simulink model "test03"  (R2022a)
settings: Solver=FixedStepAuto  StartTime=0.0  StopTime=0.05  FixedStep=1e-4  RelTol=1e-3
values from neighbouring files (symbols this model uses):
  A = 0.01    [parameter_load3.m]
  d = 2.6    [parameter_load3.m]
  I = 3055e-8    (= 3.055e-05)    [parameter_load3.m]
  jv = 1064400    [parameter_load3.m]
  kf = 6.67e7    (= 66700000)    [parameter_load3.m]
  kv = 400000    [parameter_load3.m]
  L = 210    [parameter_load3.m]
  m = 7800*(7684e-6)    (= 59.9352)    [parameter_load3.m]
  mv = 42400    [parameter_load3.m]
  position = 0.5*L    (= 105)    [parameter_load3.m]
  r = 840/1000/2    (= 0.42)    [parameter_load3.m]
  v = 100/3.6    (= 27.7777777778)    [parameter_load3.m]
(part 1 of 73)
settings Model: Creator=Spicydel  LastModifiedBy=Spicydel

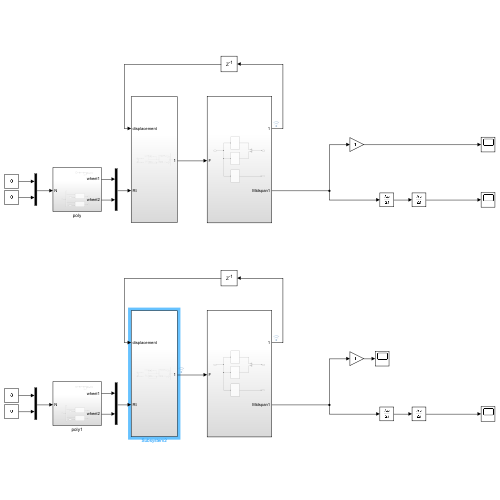
[thumbnail: Simulink's stored preview of the top-level diagram]
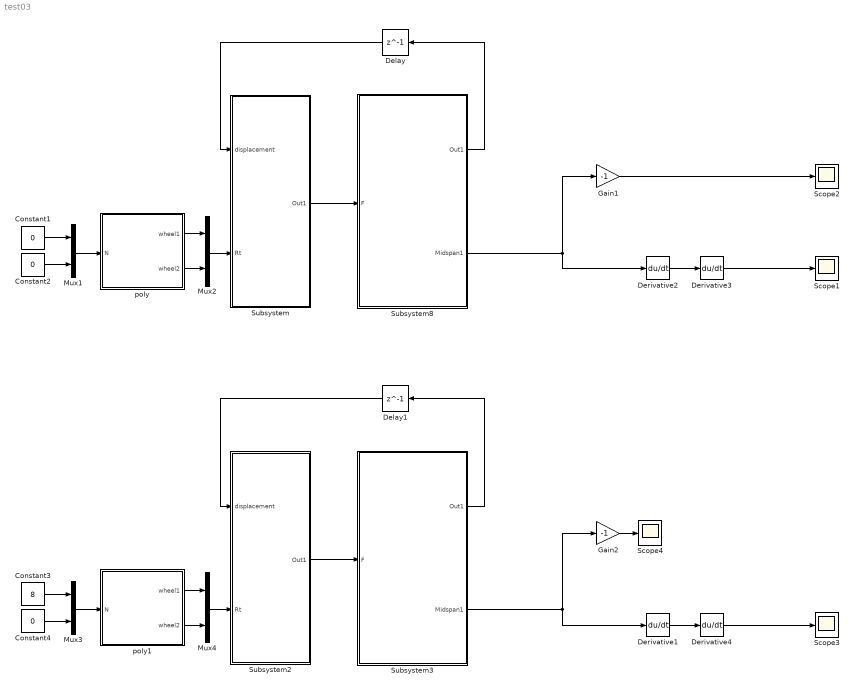
== system test03 ==
  Constant1 : Constant  Value=0
  Constant2 : Constant  Value=0
  Constant3 : Constant  Value=8
  Constant4 : Constant  Value=0
  Delay : Delay  DelayLength=1
  Delay1 : Delay  DelayLength=1
  Derivative{1..4} (4 alike) : Derivative
  Gain1 : Gain  Gain=-1
  Gain2 : Gain  Gain=-1
  Mux{1..4} (4 alike) : Mux  Inputs=2
  Scope{1..4} (4 alike) : Scope
  Subsystem : Subsystem
  Subsystem2 : Subsystem
  Subsystem3 : Subsystem
  Subsystem8 : Subsystem
  poly : Subsystem
  poly1 : Subsystem
  wires:
  Subsystem8/1(Out1) -> Delay/1
  Subsystem/1(Out1) -> Subsystem8/1(F)
  Delay/1 -> Subsystem/1(displacement)
  Mux1/1 -> poly/1(N)
  Constant1/1 -> Mux1/1
  Constant2/1 -> Mux1/2
  poly/1(wheel1) -> Mux2/1
  poly/2(wheel2) -> Mux2/2
  Mux2/1 -> Subsystem/2(Rt)
  Subsystem8/2(Midspan1) -> Derivative2/1, Gain1/1
  Derivative2/1 -> Derivative3/1
  Derivative3/1 -> Scope1/1
  Gain1/1 -> Scope2/1
  Gain2/1 -> Scope4/1
  Derivative4/1 -> Scope3/1
  Derivative1/1 -> Derivative4/1
  Subsystem3/2(Midspan1) -> Gain2/1, Derivative1/1
  Mux4/1 -> Subsystem2/2(Rt)
  poly1/2(wheel2) -> Mux4/2
  poly1/1(wheel1) -> Mux4/1
  Constant4/1 -> Mux3/2
  Constant3/1 -> Mux3/1
  Mux3/1 -> poly1/1(N)
  Delay1/1 -> Subsystem2/1(displacement)
  Subsystem2/1(Out1) -> Subsystem3/1(F)
  Subsystem3/1(Out1) -> Delay1/1

== system test03/Subsystem ==
  displacement : Inport
  Rt : Inport  Port=2
  Add : Sum
  Add1 : Sum  Inputs=+-
  Add2 : Sum  Inputs=+-
  Add3 : Sum  Inputs=+-
  Add4 : Sum  Inputs=++-
  Add5 : Sum  Inputs=+--
  Add6 : Sum  Inputs=+--
  Add7 : Sum  Inputs=+--
  Add8 : Sum
  Add9 : Sum
  Constant : Constant  Value=mv*9.8/2
  Constant2 : Constant  Value=mv*9.8/2
  Demux : Demux  Outputs=2
  Demux1 : Demux  Outputs=2
  Gain : Gain  Gain=kv/mv
  Gain1 : Gain  Gain=2*kv/mv
  Gain2 : Gain  Gain=d*kv/jv/2
  Gain3 : Gain  Gain=d*d*kv/jv/2
  Gain4 : Gain  Gain=d/2
  Gain5 : Gain  Gain=kv
  Gain6 : Gain  Gain=d/2
  Gain7 : Gain  Gain=kv
  Integrator{,1..3} (4 alike) : Integrator
  Mux : Mux  Inputs=2
  Scope : Scope  NumInputPorts=2
  Out1 : Outport
  wires:
  Mux/1 -> Out1/1
  displacement/1 -> Demux/1
  Integrator/1 -> Integrator1/1  "y_dot"
  Integrator2/1 -> Integrator3/1  "theta_dot"
  Add/1 -> Gain/1
  Integrator1/1 -> Gain1/1, Add4/1, Add5/1  "y"
  Add1/1 -> Integrator/1  "y_dot_dot"
  Gain/1 -> Add1/1
  Gain1/1 -> Add1/2
  Add2/1 -> Gain2/1
  Gain2/1 -> Add3/1
  Add3/1 -> Integrator2/1  "theta_dot_dot"
  Integrator3/1 -> Gain3/1, Gain6/1, Gain4/1
  Gain3/1 -> Add3/2
  Add4/1 -> Gain5/1
  Add5/1 -> Gain7/1  "p2"
  Demux/1 -> Add8/1  "u1"
  Demux/2 -> Add9/1  "u2"
  [u1] -> Add/1
  [u2] -> Add/2
  [u1] -> Add2/1
  [u2] -> Add2/2
  Constant/1 -> Add6/2
  Gain4/1 -> Add4/2
  [u1] -> Add4/3
  Gain5/1 -> Add6/1
  Add6/1 -> Scope/1, Mux/1  "pk1"
  Add7/1 -> Scope/2, Mux/2  "pk2"
  Constant2/1 -> Add7/2
  Gain6/1 -> Add5/2
  Gain7/1 -> Add7/1
  [u2] -> Add5/3
  Rt/1 -> Demux1/1
  Demux1/1 -> Add8/2  "u1"
  Add9/1 -> [u2]
  Demux1/2 -> Add9/2  "u2"
  Add8/1 -> [u1]

== system test03/Subsystem2 ==
  displacement : Inport
  Rt : Inport  Port=2
  Add : Sum
  Add1 : Sum  Inputs=+-
  Add2 : Sum  Inputs=+-
  Add3 : Sum  Inputs=+-
  Add4 : Sum  Inputs=++-
  Add5 : Sum  Inputs=+--
  Add6 : Sum  Inputs=+--
  Add7 : Sum  Inputs=+--
  Add8 : Sum
  Add9 : Sum
  Constant : Constant  Value=mv*9.8/2
  Constant2 : Constant  Value=mv*9.8/2
  Demux : Demux  Outputs=2
  Demux1 : Demux  Outputs=2
  Gain : Gain  Gain=kv/mv
  Gain1 : Gain  Gain=2*kv/mv
  Gain2 : Gain  Gain=d*kv/jv/2
  Gain3 : Gain  Gain=d*d*kv/jv/2
  Gain4 : Gain  Gain=d/2
  Gain5 : Gain  Gain=kv
  Gain6 : Gain  Gain=d/2
  Gain7 : Gain  Gain=kv
  Integrator{,1..3} (4 alike) : Integrator
  Mux : Mux  Inputs=2
  Scope : Scope  NumInputPorts=2
  Out1 : Outport
  wires:
  Add8/1 -> [u1]
  Demux1/2 -> Add9/2  "u2"
  Add9/1 -> [u2]
  Demux1/1 -> Add8/2  "u1"
  Rt/1 -> Demux1/1
  [u2] -> Add5/3
  Gain7/1 -> Add7/1
  Gain6/1 -> Add5/2
  Constant2/1 -> Add7/2
  Add7/1 -> Mux/2, Scope/2  "pk2"
  Add6/1 -> Mux/1, Scope/1  "pk1"
  Gain5/1 -> Add6/1
  [u1] -> Add4/3
  Gain4/1 -> Add4/2
  Constant/1 -> Add6/2
  [u2] -> Add2/2
  [u1] -> Add2/1
  [u2] -> Add/2
  [u1] -> Add/1
  Demux/2 -> Add9/1  "u2"
  Demux/1 -> Add8/1  "u1"
  Add5/1 -> Gain7/1  "p2"
  Add4/1 -> Gain5/1
  Gain3/1 -> Add3/2
  Integrator3/1 -> Gain4/1, Gain6/1, Gain3/1
  Add3/1 -> Integrator2/1  "theta_dot_dot"
  Gain2/1 -> Add3/1
  Add2/1 -> Gain2/1
  Gain1/1 -> Add1/2
  Gain/1 -> Add1/1
  Add1/1 -> Integrator/1  "y_dot_dot"
  Integrator1/1 -> Add5/1, Add4/1, Gain1/1  "y"
  Add/1 -> Gain/1
  Integrator2/1 -> Integrator3/1  "theta_dot"
  Integrator/1 -> Integrator1/1  "y_dot"
  displacement/1 -> Demux/1
  Mux/1 -> Out1/1

== system test03/Subsystem3 ==
  F : Inport
  Mux : Mux  Inputs=2
  Subsystem : Subsystem
  Subsystem1 : Subsystem
  Subsystem3 : Subsystem
  Out1 : Outport
  Midspan1 : Outport  Port=2
  wires:
  Subsystem3/1(Midspan) -> Midspan1/1
  F/1 -> Subsystem1/1(F), Subsystem3/1(F), Subsystem/1(F)
  Subsystem1/1(u2) -> Mux/2
  Mux/1 -> Out1/1
  Subsystem/1(u1) -> Mux/1

== system test03/Subsystem8 ==
  F : Inport
  Mux : Mux  Inputs=2
  Subsystem : Subsystem
  Subsystem1 : Subsystem
  Subsystem3 : Subsystem
  Out1 : Outport
  Midspan1 : Outport  Port=2
  wires:
  Subsystem/1(u1) -> Mux/1
  Mux/1 -> Out1/1
  Subsystem1/1(u2) -> Mux/2
  F/1 -> Subsystem/1(F), Subsystem3/1(F), Subsystem1/1(F)
  Subsystem3/1(Midspan) -> Midspan1/1

== system test03/poly ==
  N : Inport
  Clock : Clock
  Constant : Constant  Value=v
  Demux : Demux  Outputs=2
  Gain : Gain  Gain=1/3.6
  Gain1 : Gain  Gain=1/r
  Product1 : Product
  Subsystem : Subsystem
  Subsystem1 : Subsystem
  wheel1 : Outport
  wheel2 : Outport  Port=2
  wires:
  Constant/1 -> Gain/1
  Gain/1 -> Gain1/1
  Gain1/1 -> Product1/1
  Clock/1 -> Product1/2
  Product1/1 -> [omega]
  [omega] -> Subsystem/2(In2)
  Subsystem/1(Out1) -> wheel1/1
  [omega] -> Subsystem1/2(In2)
  Subsystem1/1(Out1) -> wheel2/1
  N/1 -> Demux/1
  Demux/1 -> Subsystem/1(In1)
  Demux/2 -> Subsystem1/1(In1)

== system test03/poly1 ==
  N : Inport
  Clock : Clock
  Constant : Constant  Value=v
  Demux : Demux  Outputs=2
  Gain : Gain  Gain=1/3.6
  Gain1 : Gain  Gain=1/r
  Product1 : Product
  Subsystem : Subsystem
  Subsystem1 : Subsystem
  wheel1 : Outport
  wheel2 : Outport  Port=2
  wires:
  Demux/2 -> Subsystem1/1(In1)
  Demux/1 -> Subsystem/1(In1)
  N/1 -> Demux/1
  Subsystem1/1(Out1) -> wheel2/1
  [omega] -> Subsystem1/2(In2)
  Subsystem/1(Out1) -> wheel1/1
  [omega] -> Subsystem/2(In2)
  Product1/1 -> [omega]
  Clock/1 -> Product1/2
  Gain1/1 -> Product1/1
  Gain/1 -> Gain1/1
  Constant/1 -> Gain/1

== system test03/Subsystem3/Subsystem ==
  F : Inport
  Add : Sum  Inputs=+++++
  Constant1 : Constant  Value=21
  Constant11 : Constant
  Constant2 : Constant  Value=41
  Constant3 : Constant  Value=61
  Constant4 : Constant  Value=81
  Subsystem : Subsystem
  Subsystem1 : Subsystem
  Subsystem2 : Subsystem
  Subsystem3 : Subsystem
  Subsystem4 : Subsystem
  u1 : Outport
  wires:
  Add/1 -> u1/1
  Subsystem4/1(Out1) -> Add/5
  Subsystem3/1(Out1) -> Add/4
  Subsystem2/1(Out1) -> Add/3
  Subsystem1/1(Out1) -> Add/2
  Subsystem/1(Out1) -> Add/1
  [Ff] -> Subsystem4/2(In2)
  Constant4/1 -> Subsystem4/1(In1)
  Constant3/1 -> Subsystem3/1(In1)
  [Ff] -> Subsystem3/2(In2)
  [Ff] -> Subsystem2/2(In2)
  Constant2/1 -> Subsystem2/1(In1)
  Constant1/1 -> Subsystem1/1(In1)
  [Ff] -> Subsystem1/2(In2)
  [Ff] -> Subsystem/2(In2)
  Constant11/1 -> Subsystem/1(In1)
  F/1 -> [Ff]

== system test03/Subsystem3/Subsystem1 ==
  F : Inport
  Add : Sum  Inputs=++++++++++++++++++++
  Add Constant{,1..18} (19 alike) : Bias  Bias=1  SaturateOnIntegerOverflow=off
  Constant11 : Constant
  Subsystem1 : Subsystem
  Subsystem10 : Subsystem
  Subsystem11 : Subsystem
  Subsystem12 : Subsystem
  Subsystem13 : Subsystem
  Subsystem14 : Subsystem
  Subsystem15 : Subsystem
  Subsystem16 : Subsystem
  Subsystem17 : Subsystem
  Subsystem18 : Subsystem
  Subsystem19 : Subsystem
  Subsystem2 : Subsystem
  Subsystem20 : Subsystem
  Subsystem3 : Subsystem
  Subsystem4 : Subsystem
  Subsystem5 : Subsystem
  Subsystem6 : Subsystem
  Subsystem7 : Subsystem
  Subsystem8 : Subsystem
  Subsystem9 : Subsystem
  u2 : Outport
  wires:
  Add Constant18/1 -> Subsystem18/1(index)
  Add Constant16/1 -> Add Constant17/1, Subsystem20/1(index)
  Add Constant17/1 -> Add Constant12/1, Subsystem12/1(index)
  Add Constant12/1 -> Add Constant13/1, Subsystem19/1(index)
  Add Constant13/1 -> Subsystem11/1(index), Add Constant18/1
  Add Constant15/1 -> Add Constant16/1, Subsystem14/1(index)
  Add Constant14/1 -> Add Constant15/1, Subsystem13/1(index)
  Add Constant11/1 -> Subsystem15/1(index), Add Constant14/1
  Add Constant5/1 -> Subsystem10/1(index), Add Constant6/1
  Add Constant6/1 -> Add Constant7/1, Subsystem8/1(index)
  Add Constant7/1 -> Add Constant8/1, Subsystem1/1(index)
  Add Constant10/1 -> Add Constant11/1, Subsystem17/1(index)
  Add Constant9/1 -> Add Constant10/1, Subsystem16/1(index)
  Add Constant8/1 -> Subsystem7/1(index), Add Constant9/1
  ? -> Add Constant3/1
  Add Constant3/1 -> Add Constant4/1, Subsystem3/1(index)
  Add Constant4/1 -> Add Constant5/1, Subsystem9/1(index)
  Add Constant2/1 -> Subsystem2/1(index)
  Add Constant1/1 -> Add Constant2/1, Subsystem4/1(index)
  Add Constant/1 -> Add Constant1/1, Subsystem6/1(index)
  Subsystem1/1(Out1) -> Add/9
  Subsystem7/1(Out1) -> Add/10
  [Ff] -> Subsystem3/2(F)
  [Ff] -> Subsystem15/2(F)
  [Ff] -> Subsystem4/2(F)
  [Ff] -> Subsystem1/2(F)
  F/1 -> [Ff]
  Subsystem20/1(Out1) -> Add/16
  [Ff] -> Subsystem20/2(F)
  Subsystem10/1(Out1) -> Add/7
  Subsystem8/1(Out1) -> Add/8
  [Ff] -> Subsystem18/2(F)
  [Ff] -> Subsystem14/2(F)
  Subsystem15/1(Out1) -> Add/13
  Subsystem19/1(Out1) -> Add/18
  Subsystem13/1(Out1) -> Add/14
  [Ff] -> Subsystem13/2(F)
  Subsystem4/1(Out1) -> Add/3
  Subsystem14/1(Out1) -> Add/15
  Subsystem16/1(Out1) -> Add/11
  Subsystem6/1(Out1) -> Add/2
  [Ff] -> Subsystem7/2(F)
  Add/1 -> u2/1
  [Ff] -> Subsystem16/2(F)
  Subsystem9/1(Out1) -> Add/6
  Subsystem17/1(Out1) -> Add/12
  [Ff] -> Subsystem11/2(F)
  [Ff] -> Subsystem8/2(F)
  Subsystem11/1(Out1) -> Add/19
  [Ff] -> Subsystem10/2(F)
  [Ff] -> Subsystem9/2(F)
  Subsystem12/1(Out1) -> Add/17
  Subsystem18/1(Out1) -> Add/20
  [Ff] -> Subsystem19/2(F)
  [Ff] -> Subsystem17/2(F)
  [Ff] -> Subsystem5/2(F)
  [Ff] -> Subsystem12/2(F)
  Subsystem2/1(Out1) -> Add/4
  [Ff] -> Subsystem6/2(F)
  Subsystem5/1(Out1) -> Add/1
  Subsystem3/1(Out1) -> Add/5
  Constant11/1 -> Subsystem5/1(index), Add Constant/1
  [Ff] -> Subsystem2/2(F)

== system test03/Subsystem3/Subsystem3 ==
  F : Inport
  Add : Sum  Inputs=+++++
  Constant1 : Constant  Value=21
  Constant11 : Constant
  Constant2 : Constant  Value=41
  Constant3 : Constant  Value=61
  Constant4 : Constant  Value=81
  Subsystem : Subsystem
  Subsystem1 : Subsystem
  Subsystem2 : Subsystem
  Subsystem3 : Subsystem
  Subsystem4 : Subsystem
  Midspan : Outport
  wires:
  Subsystem4/1(Out1) -> Add/5
  Subsystem3/1(Out1) -> Add/4
  [Ff] -> Subsystem4/1(In2)
  Constant4/1 -> Subsystem4/2(In1)
  Constant3/1 -> Subsystem3/2(In1)
  [Ff] -> Subsystem3/1(In2)
  [Ff] -> Subsystem2/1(In2)
  [Ff] -> Subsystem1/1(In2)
  [Ff] -> Subsystem/1(In2)
  Add/1 -> Midspan/1
  Subsystem2/1(Out1) -> Add/3
  Constant2/1 -> Subsystem2/2(In1)
  Subsystem1/1(Out1) -> Add/2
  Constant1/1 -> Subsystem1/2(In1)
  Subsystem/1(Out1) -> Add/1
  Constant11/1 -> Subsystem/2(In1)
  F/1 -> [Ff]

== system test03/Subsystem8/Subsystem ==
  F : Inport
  Add : Sum  Inputs=+++++
  Constant1 : Constant  Value=21
  Constant11 : Constant
  Constant2 : Constant  Value=41
  Constant3 : Constant  Value=61
  Constant4 : Constant  Value=81
  Subsystem : Subsystem
  Subsystem1 : Subsystem
  Subsystem2 : Subsystem
  Subsystem3 : Subsystem
  Subsystem4 : Subsystem
  u1 : Outport
  wires:
  F/1 -> [Ff]
  Constant11/1 -> Subsystem/1(In1)
  [Ff] -> Subsystem/2(In2)
  [Ff] -> Subsystem1/2(In2)
  Constant1/1 -> Subsystem1/1(In1)
  Constant2/1 -> Subsystem2/1(In1)
  [Ff] -> Subsystem2/2(In2)
  [Ff] -> Subsystem3/2(In2)
  Constant3/1 -> Subsystem3/1(In1)
  Constant4/1 -> Subsystem4/1(In1)
  [Ff] -> Subsystem4/2(In2)
  Subsystem/1(Out1) -> Add/1
  Subsystem1/1(Out1) -> Add/2
  Subsystem2/1(Out1) -> Add/3
  Subsystem3/1(Out1) -> Add/4
  Subsystem4/1(Out1) -> Add/5
  Add/1 -> u1/1

== system test03/Subsystem8/Subsystem1 ==
  F : Inport
  Add : Sum  Inputs=++++++++++++++++++++
  Add Constant{,1..18} (19 alike) : Bias  Bias=1  SaturateOnIntegerOverflow=off
  Constant11 : Constant
  Subsystem1 : Subsystem
  Subsystem10 : Subsystem
  Subsystem11 : Subsystem
  Subsystem12 : Subsystem
  Subsystem13 : Subsystem
  Subsystem14 : Subsystem
  Subsystem15 : Subsystem
  Subsystem16 : Subsystem
  Subsystem17 : Subsystem
  Subsystem18 : Subsystem
  Subsystem19 : Subsystem
  Subsystem2 : Subsystem
  Subsystem20 : Subsystem
  Subsystem3 : Subsystem
  Subsystem4 : Subsystem
  Subsystem5 : Subsystem
  Subsystem6 : Subsystem
  Subsystem7 : Subsystem
  Subsystem8 : Subsystem
  Subsystem9 : Subsystem
  u2 : Outport
  wires:
  [Ff] -> Subsystem2/2(F)
  Constant11/1 -> Add Constant/1, Subsystem5/1(index)
  Subsystem3/1(Out1) -> Add/5
  Subsystem5/1(Out1) -> Add/1
  [Ff] -> Subsystem6/2(F)
  Subsystem2/1(Out1) -> Add/4
  [Ff] -> Subsystem12/2(F)
  [Ff] -> Subsystem5/2(F)
  [Ff] -> Subsystem17/2(F)
  [Ff] -> Subsystem19/2(F)
  Subsystem18/1(Out1) -> Add/20
  Subsystem12/1(Out1) -> Add/17
  [Ff] -> Subsystem9/2(F)
  [Ff] -> Subsystem10/2(F)
  Subsystem11/1(Out1) -> Add/19
  [Ff] -> Subsystem8/2(F)
  [Ff] -> Subsystem11/2(F)
  Subsystem17/1(Out1) -> Add/12
  Subsystem9/1(Out1) -> Add/6
  [Ff] -> Subsystem16/2(F)
  Add/1 -> u2/1
  [Ff] -> Subsystem7/2(F)
  Subsystem6/1(Out1) -> Add/2
  Subsystem16/1(Out1) -> Add/11
  Subsystem14/1(Out1) -> Add/15
  Subsystem4/1(Out1) -> Add/3
  [Ff] -> Subsystem13/2(F)
  Subsystem13/1(Out1) -> Add/14
  Subsystem19/1(Out1) -> Add/18
  Subsystem15/1(Out1) -> Add/13
  [Ff] -> Subsystem14/2(F)
  [Ff] -> Subsystem18/2(F)
  Subsystem8/1(Out1) -> Add/8
  Subsystem10/1(Out1) -> Add/7
  [Ff] -> Subsystem20/2(F)
  Subsystem20/1(Out1) -> Add/16
  F/1 -> [Ff]
  [Ff] -> Subsystem1/2(F)
  [Ff] -> Subsystem4/2(F)
  [Ff] -> Subsystem15/2(F)
  [Ff] -> Subsystem3/2(F)
  Subsystem7/1(Out1) -> Add/10
  Subsystem1/1(Out1) -> Add/9
  Add Constant/1 -> Subsystem6/1(index), Add Constant1/1
  Add Constant1/1 -> Subsystem4/1(index), Add Constant2/1
  Add Constant2/1 -> Subsystem2/1(index)
  Add Constant4/1 -> Subsystem9/1(index), Add Constant5/1
  Add Constant3/1 -> Subsystem3/1(index), Add Constant4/1
  ? -> Add Constant3/1
  Add Constant8/1 -> Add Constant9/1, Subsystem7/1(index)
  Add Constant9/1 -> Subsystem16/1(index), Add Constant10/1
  Add Constant10/1 -> Subsystem17/1(index), Add Constant11/1
  Add Constant7/1 -> Subsystem1/1(index), Add Constant8/1
  Add Constant6/1 -> Subsystem8/1(index), Add Constant7/1
  Add Constant5/1 -> Add Constant6/1, Subsystem10/1(index)
  Add Constant11/1 -> Add Constant14/1, Subsystem15/1(index)
  Add Constant14/1 -> Subsystem13/1(index), Add Constant15/1
  Add Constant15/1 -> Subsystem14/1(index), Add Constant16/1
  Add Constant13/1 -> Add Constant18/1, Subsystem11/1(index)
  Add Constant12/1 -> Subsystem19/1(index), Add Constant13/1
  Add Constant17/1 -> Subsystem12/1(index), Add Constant12/1
  Add Constant16/1 -> Subsystem20/1(index), Add Constant17/1
  Add Constant18/1 -> Subsystem18/1(index)

== system test03/Subsystem8/Subsystem3 ==
  F : Inport
  Add : Sum  Inputs=+++++
  Constant1 : Constant  Value=21
  Constant11 : Constant
  Constant2 : Constant  Value=41
  Constant3 : Constant  Value=61
  Constant4 : Constant  Value=81
  Subsystem : Subsystem
  Subsystem1 : Subsystem
  Subsystem2 : Subsystem
  Subsystem3 : Subsystem
  Subsystem4 : Subsystem
  Midspan : Outport
  wires:
  F/1 -> [Ff]
  Constant11/1 -> Subsystem/2(In1)
  Subsystem/1(Out1) -> Add/1
  Constant1/1 -> Subsystem1/2(In1)
  Subsystem1/1(Out1) -> Add/2
  Constant2/1 -> Subsystem2/2(In1)
  Subsystem2/1(Out1) -> Add/3
  Add/1 -> Midspan/1
  [Ff] -> Subsystem/1(In2)
  [Ff] -> Subsystem1/1(In2)
  [Ff] -> Subsystem2/1(In2)
  [Ff] -> Subsystem3/1(In2)
  Constant3/1 -> Subsystem3/2(In1)
  Constant4/1 -> Subsystem4/2(In1)
  [Ff] -> Subsystem4/1(In2)
  Subsystem3/1(Out1) -> Add/4
  Subsystem4/1(Out1) -> Add/5

== system test03/poly/Subsystem ==
  In1 : Inport
  In2 : Inport  Port=2
  Add : Sum
  Add1 : Sum
  Add2 : Sum  Inputs=+-
  Constant : Constant  Value=r
  Constant1 : Constant  Value=r
  Constant2 : Constant  Value=0
  Cos : Trigonometry  Operator=cos
  Gain2 : Gain  Gain=A
  Product : Product
  Product2 : Product
  Product3 : Product
  Sin : Trigonometry
  Sin1 : Trigonometry
  Sqrt : Sqrt
  Square : Math  Operator=square
  Square1 : Math  Operator=square
  Switch : Switch  Criteria=u2 ~= 0  SaturateOnIntegerOverflow=off  InputSameDT=off
  Out1 : Outport
  wires:
  Cos/1 -> Product3/2
  Product/1 -> Sin/1
  Sin/1 -> Gain2/1
  Gain2/1 -> Add/1
  Add/1 -> Product3/1, Product2/2
  Constant/1 -> Add/2
  [omega] -> Sin1/1
  Sin1/1 -> Product2/1
  [omega] -> Cos/1
  Product2/1 -> Square/1
  Constant1/1 -> Add2/2
  Sqrt/1 -> Add2/1
  Square1/1 -> Add1/2
  Product3/1 -> Square1/1
  Square/1 -> Add1/1
  Add1/1 -> Sqrt/1
  In2/1 -> [omega], Product/2
  [N] -> Product/1
  In1/1 -> [N]
  [N] -> Switch/2
  Switch/1 -> Out1/1
  Add2/1 -> Switch/1
  Constant2/1 -> Switch/3

== system test03/poly/Subsystem1 ==
  In1 : Inport
  In2 : Inport  Port=2
  Add : Sum
  Add1 : Sum
  Add2 : Sum  Inputs=+-
  Constant : Constant  Value=r
  Constant1 : Constant  Value=r
  Constant2 : Constant  Value=0
  Cos : Trigonometry  Operator=cos
  Gain2 : Gain  Gain=A
  Product : Product
  Product2 : Product
  Product3 : Product
  Sin : Trigonometry
  Sin1 : Trigonometry
  Sqrt : Sqrt
  Square : Math  Operator=square
  Square1 : Math  Operator=square
  Switch : Switch  Criteria=u2 ~= 0  SaturateOnIntegerOverflow=off  InputSameDT=off
  Out1 : Outport
  wires:
  Constant2/1 -> Switch/3
  Add2/1 -> Switch/1
  Switch/1 -> Out1/1
  [N] -> Switch/2
  In1/1 -> [N]
  [N] -> Product/1
  In2/1 -> Product/2, [omega]
  Add1/1 -> Sqrt/1
  Square/1 -> Add1/1
  Product3/1 -> Square1/1
  Square1/1 -> Add1/2
  Sqrt/1 -> Add2/1
  Constant1/1 -> Add2/2
  Product2/1 -> Square/1
  [omega] -> Cos/1
  Sin1/1 -> Product2/1
  [omega] -> Sin1/1
  Constant/1 -> Add/2
  Add/1 -> Product2/2, Product3/1
  Gain2/1 -> Add/1
  Sin/1 -> Gain2/1
  Product/1 -> Sin/1
  Cos/1 -> Product3/2

== system test03/poly1/Subsystem ==
  In1 : Inport
  In2 : Inport  Port=2
  Add : Sum
  Add1 : Sum
  Add2 : Sum  Inputs=+-
  Constant : Constant  Value=r
  Constant1 : Constant  Value=r
  Constant2 : Constant  Value=0
  Cos : Trigonometry  Operator=cos
  Gain2 : Gain  Gain=A
  Product : Product
  Product2 : Product
  Product3 : Product
  Sin : Trigonometry
  Sin1 : Trigonometry
  Sqrt : Sqrt
  Square : Math  Operator=square
  Square1 : Math  Operator=square
  Switch : Switch  Criteria=u2 ~= 0  SaturateOnIntegerOverflow=off  InputSameDT=off
  Out1 : Outport
  wires:
  Constant2/1 -> Switch/3
  Add2/1 -> Switch/1
  Switch/1 -> Out1/1
  [N] -> Switch/2
  In1/1 -> [N]
  [N] -> Product/1
  In2/1 -> Product/2, [omega]
  Add1/1 -> Sqrt/1
  Square/1 -> Add1/1
  Product3/1 -> Square1/1
  Square1/1 -> Add1/2
  Sqrt/1 -> Add2/1
  Constant1/1 -> Add2/2
  Product2/1 -> Square/1
  [omega] -> Cos/1
  Sin1/1 -> Product2/1
  [omega] -> Sin1/1
  Constant/1 -> Add/2
  Add/1 -> Product2/2, Product3/1
  Gain2/1 -> Add/1
  Sin/1 -> Gain2/1
  Product/1 -> Sin/1
  Cos/1 -> Product3/2

== system test03/poly1/Subsystem1 ==
  In1 : Inport
  In2 : Inport  Port=2
  Add : Sum
  Add1 : Sum
  Add2 : Sum  Inputs=+-
  Constant : Constant  Value=r
  Constant1 : Constant  Value=r
  Constant2 : Constant  Value=0
  Cos : Trigonometry  Operator=cos
  Gain2 : Gain  Gain=A
  Product : Product
  Product2 : Product
  Product3 : Product
  Sin : Trigonometry
  Sin1 : Trigonometry
  Sqrt : Sqrt
  Square : Math  Operator=square
  Square1 : Math  Operator=square
  Switch : Switch  Criteria=u2 ~= 0  SaturateOnIntegerOverflow=off  InputSameDT=off
  Out1 : Outport
  wires:
  Cos/1 -> Product3/2
  Product/1 -> Sin/1
  Sin/1 -> Gain2/1
  Gain2/1 -> Add/1
  Add/1 -> Product3/1, Product2/2
  Constant/1 -> Add/2
  [omega] -> Sin1/1
  Sin1/1 -> Product2/1
  [omega] -> Cos/1
  Product2/1 -> Square/1
  Constant1/1 -> Add2/2
  Sqrt/1 -> Add2/1
  Square1/1 -> Add1/2
  Product3/1 -> Square1/1
  Square/1 -> Add1/1
  Add1/1 -> Sqrt/1
  In2/1 -> [omega], Product/2
  [N] -> Product/1
  In1/1 -> [N]
  [N] -> Switch/2
  Switch/1 -> Out1/1
  Add2/1 -> Switch/1
  Constant2/1 -> Switch/3
